simulink model "ofdm_txrx_supermimo_coded"  (Simulink 7.7)
settings: Solver=VariableStepDiscrete  SolverMode=SingleTasking  StartTime=0.0  StopTime=2*simOnly_simLength  RelTol=1e-3
PostLoadFcn:
  | ofdm_rx_supermimo_init
InitFcn:
  | ofdm_rx_supermimo_init
StartFcn:
  | ofdm_rx_supermimo_init
values from neighbouring files (symbols this model uses):
  ActionID_AFTransmit = 31    [ofdm_tx_supermimo_init.m]
  ActionID_Disabled = 0    [ofdm_tx_supermimo_init.m]
  ActionID_SetFlagA = 62    [ofdm_tx_supermimo_init.m]
  ActionID_SetFlagB = 61    [ofdm_tx_supermimo_init.m]
  BER_RAM_init_size = 2048    [ofdm_tx_supermimo_init.m]
  channel_h = [1 0; 1 0] * 0.3    [ofdm_tx_supermimo_init.m]
  CPLength = 16    [ofdm_tx_supermimo_init.m]
  longCorr_coef_i = [-4*real(LTS) 0]    [ofdm_rx_supermimo_init.m]
  longCorr_coef_q = [4*imag(LTS) 0]    [ofdm_rx_supermimo_init.m]
  max_CP_length = 16    [ofdm_tx_supermimo_init.m]
  max_num_baseRateSymbols = 31    [ofdm_tx_supermimo_init.m]
  max_num_subcarriers = 64    [ofdm_tx_supermimo_init.m]
  max_num_trainingSymbols = 15    [ofdm_tx_supermimo_init.m]
  max_numBytes = 16384    [ofdm_tx_supermimo_init.m]
  max_OFDM_symbols = 1023    [ofdm_tx_supermimo_init.m]
  modConstellation_qam16 = .75*[1 3 -1 -3]./3    [ofdm_tx_supermimo_init.m]
  modConstellation_qam64 = 0.875*[3 1 5 7 -3 -1 -5 -7]./7    [ofdm_tx_supermimo_init.m]
  modConstellation_qpsk = [1 -1]./sqrt(2)    [ofdm_tx_supermimo_init.m]
  numBaseRateSymbols = numBaseRateSymbols *2    [ofdm_tx_supermimo_init.m]
  numSubcarriers = 64    [ofdm_tx_supermimo_init.m]
  numTrainingSymbols = 2    [ofdm_tx_supermimo_init.m]
  pktTiming_count_payloadStart = pktTiming_count_lsStart + pktTiming_ls_num*pktTiming_ls_length    [ofdm_rx_supermimo_init.m]
  preamble = preamble_scale*[shortsyms_10 longsyms_2]    [ofdm_tx_supermimo_init.m]
  preamble_ROM = preamble_I_ROM + preamble_Q_ROM*2^16    [ofdm_tx_supermimo_init.m]
  RAM_init_size = max_numBytes    (= 16384)    [ofdm_tx_supermimo_init.m]
  RxDataScrambling_Seq = [40 198 78 63 82 173 102 245 48 111 172 115 147 230 216 93 72 65 62 2 205 242 122 90 128 83 105 97 73 10 5 252]    [ofdm_tx_supermimo_init.m]
  shortSymbol_time = shortSymbol_time(1:16).'    [ofdm_tx_supermimo_init.m]
  simOnly_numSamples = length(preamble)+( (numSubcarriers+CPLength)*(numTrainingSymbols + numBaseRateSymbols + numFullRateSymbols/2) )    [ofdm_tx_supermimo_init.m]
  train = [train train]    [ofdm_tx_supermimo_init.m]
  TxDataScrambling_Seq = [40 198 78 63 82 173 102 245 48 111 172 115 147 230 216 93 72 65 62 2 205 242 122 90 128 83 105 97 73 10 5 252]    [ofdm_tx_supermimo_init.m]
undefined symbols (not defined in the file or in its folder): AntA, AntB, BaseRate, Down, gcbh, numBuffs, numBytes, Up, xlBlockLoadCallback, xlGetNormalizedPeriod, xlipgetpartsetting
libraries: xbsIndex_r4 x5430
(part 36 of 49)

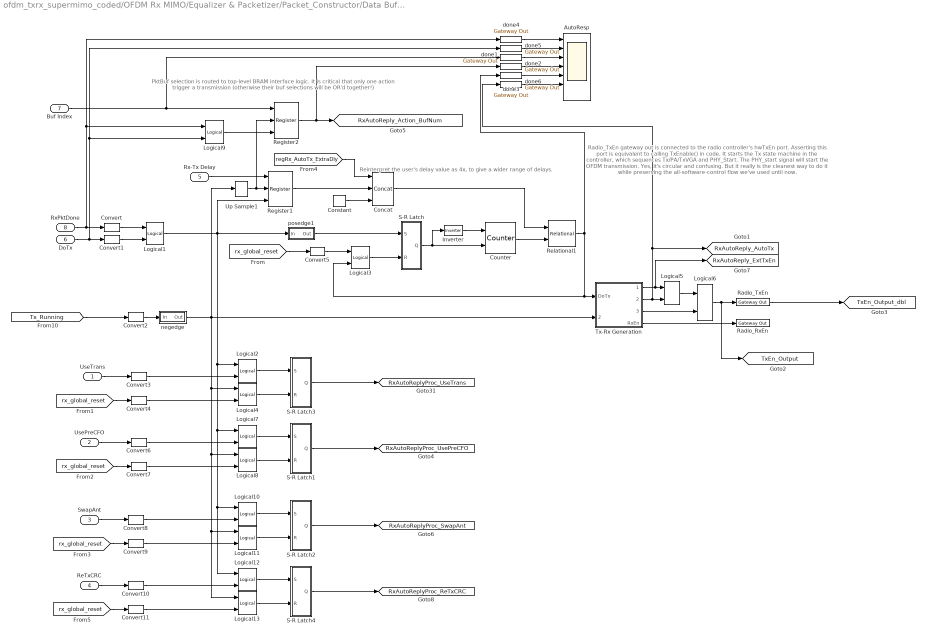
== system ofdm_txrx_supermimo_coded/OFDM Rx MIMO/Equalizer & Packetizer/Packet_Constructor/Data Buffer/AutoResponse Processing/AutoResponse I/O ==
  note: Radio_TxEn gateway out is connected to the radio controller's hwTxEn port.
      | Asserting this port is equivalent to calling TxEnable() in code. It starts the Tx
      | state machine in the controller, which sequences Tx/PA/TxVGA and PHY_Start.
      | The PHY_start signal will start the OFDM transmission.
      |
      | Yes, it's circular and confusing. But it really is the cleanest way to do it while
      | preserving the all-software-control flow we've used until now.
  note: PktBuf selection is routed to top-level
      | BRAM interface logic. It is critical that only
      | one action trigger a transmission (otherwise
      | their buf selections will be OR'd together!)
  note: Reinterpret the user's delay value as 4x,
      | to give a wider range of delays.
  UseTrans : Inport
  UsePreCFO : Inport  Port=2
  SwapAnt : Inport  Port=3
  ReTxCRC : Inport  Port=4
  Rx-Tx Delay : Inport  Port=5
  DoTx : Inport  Port=6
  Buf Index : Inport  Port=7
  RxPktDone : Inport  Port=8
  AutoResp : Scope  NumInputPorts=7
      settings: TimeRange=392.7801724137931  YMin=-0.5~-0.25~-1~-1~0~0~0  YMax=0.5~0.15~1~1~1~3500~1  SaveName=ScopeData25
  Concat : xbsIndex_r4/Concat  num_inputs=3  dbl_ovrd=off  block_type=concat
      settings: block_version=10.1.3
  Constant : xbsIndex_r4/Constant  const=0  gui_display_data_type=Fixed-point  arith_type=Unsigned  n_bits=1  bin_pt=0  preci_type=Single  exp_width=8  frac_width=24  explicit_period=off  period=1  equ=P=C  opselect=C  inp2=PCIN>>17  opr=+  inp1=P  carry=CIN  dbl_ovrd=off  block_type=constant
  Convert{,1..11} (12 alike) : xbsIndex_r4/Convert  gui_display_data_type=Fixed-point  arith_type=Boolean  n_bits=16  bin_pt=14  float_type=Single  exp_bits=8  fraction_bits=24  quantization=Truncate  overflow=Wrap  en=off  latency=0  dbl_ovrd=off  pipeline=off  block_type=convert
      settings: block_version=10.1.3
  Counter : xbsIndex_r4/Counter  cnt_type=Free Running  cnt_to=0  operation=Up  start_count=0  cnt_by_val=1  arith_type=Unsigned  n_bits=14  bin_pt=0  load_pin=off  rst=on  en=on  explicit_period=off  period=4  dbl_ovrd=off  use_behavioral_HDL=off  implementation=Fabric  use_rpm=off  block_type=counter
      settings: block_version=VER_STRING_GOES_HERE
  Inverter : xbsIndex_r4/Inverter  en=off  latency=0  dbl_ovrd=off  block_type=inv
      settings: xl_area=[0 0 0 0 0 0 0]  block_version=8.2
  Logical{1..2,7,9..10,12} (6 alike) : xbsIndex_r4/Logical  logical_function=AND  inputs=2  en=off  latency=0  precision=Full  arith_type=Unsigned  n_bits=16  bin_pt=0  align_bp=on  dbl_ovrd=off  block_type=logical
  Logical{3..6,8,11,13} (7 alike) : xbsIndex_r4/Logical  logical_function=OR  inputs=2  en=off  latency=0  precision=Full  arith_type=Unsigned  n_bits=16  bin_pt=0  align_bp=on  dbl_ovrd=off  block_type=logical
  Radio_RxEn : xbsIndex_r4/Gateway Out  inherit_from_input=off  hdl_port=on  timing_constraint=None  locs_specified=off  LOCs={}  UseAsDAC=off  DACChannel='1'  block_type=gatewayout
      settings: infoedit="Gateway out block.  Converts Xilinx fixed point inputs into ouputs of type Simulink integer, double, or fixed point.<P><P>Hardware notes:  In hardware these blocks become top level output ports or are discarded, depending on how they are configured."  block_version=10.1.3
  Radio_TxEn : xbsIndex_r4/Gateway Out  inherit_from_input=off  hdl_port=on  timing_constraint=None  locs_specified=off  LOCs={}  UseAsDAC=off  DACChannel='1'  block_type=gatewayout
      settings: infoedit="Gateway out block.  Converts Xilinx fixed point inputs into ouputs of type Simulink integer, double, or fixed point.<P><P>Hardware notes:  In hardware these blocks become top level output ports or are discarded, depending on how they are configured."  block_version=10.1.3
  Register1 : xbsIndex_r4/Register  init=0  rst=on  en=on  dbl_ovrd=off  block_type=register
      settings: xl_area=[5 10 0 0 0 0 0]  block_version=VER_STRING_GOES_HERE
  Register2 : xbsIndex_r4/Register  init=0  rst=on  en=on  dbl_ovrd=off  block_type=register
      settings: xl_area=[5 10 0 0 0 0 0]  block_version=VER_STRING_GOES_HERE
  Relational1 : xbsIndex_r4/Relational  mode=a<b  en=off  latency=0  dbl_ovrd=off  block_type=relational
      settings: block_version=VER_STRING_GOES_HERE
  S-R Latch : Subsystem
  S-R Latch1 : Subsystem
  S-R Latch2 : Subsystem
  S-R Latch3 : Subsystem
  S-R Latch4 : Subsystem
  Tx-Rx Generation : Subsystem
  Up Sample1 : xbsIndex_r4/Up Sample  sample_ratio=2  copy_samples=on  en=off  latency=0  dbl_ovrd=off  block_type=usamp
      settings: infoedit="Up samples input data.  Inserted values  can be zeros or copies of the most recent input sample.<P><P>Hardware notes: No hardware is needed if inserted values are copies of the input sample; otherwise, a mux and single bit flip-flop are used."  block_version=10.1.3
  done{1..6} (6 alike) : xbsIndex_r4/Gateway Out  inherit_from_input=off  hdl_port=off  timing_constraint=None  locs_specified=off  LOCs={}  UseAsDAC=off  DACChannel='1'  block_type=gatewayout
      settings: infoedit="Gateway out block.  Converts Xilinx fixed point inputs into ouputs of type Simulink integer, double, or fixed point.<P><P>Hardware notes:  In hardware these blocks become top level output ports or are discarded, depending on how they are configured."  xl_area=[0 0 0 0 1 0 0]  block_version=VER_STRING_GOES_HERE
  negedge : Subsystem
  posedge1 : Subsystem
  tag scope: RxAutoReplyProc_ReTxCRC=global, RxAutoReplyProc_SwapAnt=global, RxAutoReplyProc_UsePreCFO=global, RxAutoReplyProc_UseTrans=global, RxAutoReply_Action_BufNum=global, RxAutoReply_AutoTx=global, RxAutoReply_ExtTxEn=global, TxEn_Output=global, TxEn_Output_dbl=global
  wires:
  S-R Latch/1(Q) -> Counter/2, Inverter/1
  negedge/1(Out) -> Up Sample1/1, Logical4/1, Logical8/1, Logical11/1, Logical13/1, Tx-Rx Generation/2(TxDone)
  [Tx_Running] -> Convert2/1
  posedge1/1(Out) -> S-R Latch/1(S)
  Inverter/1 -> Counter/1
  Tx-Rx Generation/4(RxEn) -> Radio_RxEn/1
  Relational1/1 -> Logical3/2, Tx-Rx Generation/1(DoTx), done3/1
  Logical3/1 -> S-R Latch/2(R)
  [rx_global_reset] -> Convert5/1
  Logical1/1 -> posedge1/1(In), Register1/3, Logical2/1, Logical7/1, Logical10/1, Logical12/1
  Logical2/1 -> S-R Latch3/1(S)
  S-R Latch3/1(Q) -> [RxAutoReplyProc_UseTrans]
  Radio_TxEn/1 -> [TxEn_Output_dbl]
  DoTx/1 -> Convert1/1, Logical9/2, done5/1
  RxPktDone/1 -> Convert/1, Logical9/1, done4/1
  UseTrans/1 -> Convert3/1
  [rx_global_reset] -> Convert4/1
  Logical4/1 -> S-R Latch3/2(R)
  Convert/1 -> Logical1/1
  Convert1/1 -> Logical1/2
  Convert2/1 -> negedge/1(In)
  Convert3/1 -> Logical2/2
  Convert4/1 -> Logical4/2
  Convert5/1 -> Logical3/1
  Tx-Rx Generation/2(AutoTxEn) -> Logical5/2, [RxAutoReply_AutoTx], done6/1
  Logical6/1 -> Radio_TxEn/1, [TxEn_Output]
  Tx-Rx Generation/3(UserTxEn) -> Logical6/2
  Tx-Rx Generation/1(ExtTxEn) -> Logical5/1, [RxAutoReply_ExtTxEn]
  Logical5/1 -> Logical6/1
  Rx-Tx Delay/1 -> Register1/1
  Up Sample1/1 -> Register1/2, Register2/2
  Logical7/1 -> S-R Latch1/1(S)
  S-R Latch1/1(Q) -> [RxAutoReplyProc_UsePreCFO]
  UsePreCFO/1 -> Convert6/1
  [rx_global_reset] -> Convert7/1
  Logical8/1 -> S-R Latch1/2(R)
  Convert6/1 -> Logical7/2
  Convert7/1 -> Logical8/2
  Logical9/1 -> Register2/3
  Buf Index/1 -> Register2/1, done1/1
  Register2/1 -> [RxAutoReply_Action_BufNum], done2/1
  Constant/1 -> Concat/3
  Register1/1 -> Concat/2
  Concat/1 -> Relational1/1
  Counter/1 -> Relational1/2
  SwapAnt/1 -> Convert8/1
  Logical10/1 -> S-R Latch2/1(S)
  Logical11/1 -> S-R Latch2/2(R)
  Convert8/1 -> Logical10/2
  [rx_global_reset] -> Convert9/1
  Convert9/1 -> Logical11/2
  S-R Latch2/1(Q) -> [RxAutoReplyProc_SwapAnt]
  [regRx_AutoTx_ExtraDly] -> Concat/1
  done4/1 -> AutoResp/1
  done5/1 -> AutoResp/2
  done1/1 -> AutoResp/3
  done2/1 -> AutoResp/4
  done3/1 -> AutoResp/5
  done6/1 -> AutoResp/6
  ReTxCRC/1 -> Convert10/1
  Logical12/1 -> S-R Latch4/1(S)
  Logical13/1 -> S-R Latch4/2(R)
  Convert10/1 -> Logical12/2
  [rx_global_reset] -> Convert11/1
  Convert11/1 -> Logical13/2
  S-R Latch4/1(Q) -> [RxAutoReplyProc_ReTxCRC]

== system ofdm_txrx_supermimo_coded/OFDM Rx MIMO/Equalizer & Packetizer/Packet_Constructor/Data Buffer/AutoResponse Processing/Flag A ==
  note: The flag is cleared after every packet reception. The clear is
      | delayed 2 cycles so the matching logic can use the flag set by the
      | previous packet in combination with header/status values
      | from the packet that just finished.
      |
      | The flag is set 1 cycle later if some action asserts the SetFlag input.
  SetFlag : Inport
  PktDone : Inport  Port=2
  Delay : xbsIndex_r4/Delay  rst=off  infoeditControl=Selection of Reset will increase slice count due to use of real FFs and instead of SRLs  en=off  latency=2  dbl_ovrd=off  reg_retiming=off  block_type=delay
  Delay1 : xbsIndex_r4/Delay  rst=off  infoeditControl=Selection of Reset will increase slice count due to use of real FFs and instead of SRLs  en=off  latency=1  dbl_ovrd=off  reg_retiming=off  block_type=delay
  Flag A Latch : Subsystem
  Logical1 : xbsIndex_r4/Logical  logical_function=AND  inputs=2  en=off  latency=0  precision=Full  arith_type=Unsigned  n_bits=16  bin_pt=0  align_bp=on  dbl_ovrd=off  block_type=logical
  Logical2 : xbsIndex_r4/Logical  logical_function=OR  inputs=3  en=off  latency=0  precision=Full  arith_type=Unsigned  n_bits=16  bin_pt=0  align_bp=on  dbl_ovrd=off  block_type=logical
  Flag : Outport
  wires:
  [rx_global_reset] -> Logical2/2
  Logical2/1 -> Flag A Latch/2(R)
  Delay/1 -> Logical2/1, Delay1/1
  Logical1/1 -> Flag A Latch/1(S)
  Flag A Latch/1(Q) -> Flag/1
  PktDone/1 -> Delay/1
  SetFlag/1 -> Logical1/1
  Delay1/1 -> Logical1/2
  [RxReg_ResetFlagA] -> Logical2/3

== system ofdm_txrx_supermimo_coded/OFDM Rx MIMO/Equalizer & Packetizer/Packet_Constructor/Data Buffer/AutoResponse Processing/Flag B ==
  note: The flag is cleared after every packet reception. The clear is
      | delayed 2 cycles so the matching logic can use the flag set by the
      | previous packet in combination with header/status values
      | from the packet that just finished.
      |
      | The flag is set 1 cycle later if some action asserts the SetFlag input.
  SetFlag : Inport
  PktDone : Inport  Port=2
  Delay : xbsIndex_r4/Delay  rst=off  infoeditControl=Selection of Reset will increase slice count due to use of real FFs and instead of SRLs  en=off  latency=2  dbl_ovrd=off  reg_retiming=off  block_type=delay
  Delay1 : xbsIndex_r4/Delay  rst=off  infoeditControl=Selection of Reset will increase slice count due to use of real FFs and instead of SRLs  en=off  latency=1  dbl_ovrd=off  reg_retiming=off  block_type=delay
  Flag B Latch : Subsystem
  Logical1 : xbsIndex_r4/Logical  logical_function=AND  inputs=2  en=off  latency=0  precision=Full  arith_type=Unsigned  n_bits=16  bin_pt=0  align_bp=on  dbl_ovrd=off  block_type=logical
  Logical2 : xbsIndex_r4/Logical  logical_function=OR  inputs=3  en=off  latency=0  precision=Full  arith_type=Unsigned  n_bits=16  bin_pt=0  align_bp=on  dbl_ovrd=off  block_type=logical
  Flag : Outport
  wires:
  [RxReg_ResetFlagB] -> Logical2/3
  Delay1/1 -> Logical1/2
  SetFlag/1 -> Logical1/1
  PktDone/1 -> Delay/1
  Flag B Latch/1(Q) -> Flag/1
  Logical1/1 -> Flag B Latch/1(S)
  Delay/1 -> Delay1/1, Logical2/1
  Logical2/1 -> Flag B Latch/2(R)
  [rx_global_reset] -> Logical2/2

== system ofdm_txrx_supermimo_coded/OFDM Rx MIMO/Equalizer & Packetizer/Packet_Constructor/Data Buffer/AutoResponse Processing/Header Byte Matching ==
  RxByteAddr : Inport
  RxByte : Inport  Port=2
  RxByteWE : Inport  Port=3
  Rst : Inport  Port=4
  Concat : xbsIndex_r4/Concat  num_inputs=8  dbl_ovrd=off  block_type=concat
      settings: block_version=10.1.3
  Constant : xbsIndex_r4/Constant  const=0  gui_display_data_type=Fixed-point  arith_type=Unsigned  n_bits=1  bin_pt=0  preci_type=Single  exp_width=8  frac_width=24  explicit_period=off  period=1  equ=P=C  opselect=C  inp2=PCIN>>17  opr=+  inp1=P  carry=CIN  dbl_ovrd=off  block_type=constant
  Field Slices : Subsystem
  Field Slices1 : Subsystem
  Field Slices2 : Subsystem
  Field Slices3 : Subsystem
  Field Slices4 : Subsystem
  Field Slices5 : Subsystem
  Match0 : Subsystem
  Match1 : Subsystem
  Match2 : Subsystem
  Match3 : Subsystem
  Match4 : Subsystem
  Match5 : Subsystem
  Match_8b : Outport
  wires:
  [regAutoReply_match5] -> Field Slices5/1(Reg 32b)
  Field Slices5/1(Addr) -> Match5/5(Match ByteAddr)
  Field Slices5/2(Len) -> Match5/6(Match Length)
  Field Slices5/3(Val) -> Match5/7(Match Bytes)
  RxByteAddr/1 -> Match0/2(Rx ByteAddr), Match1/2(Rx ByteAddr), Match2/2(Rx ByteAddr), Match3/2(Rx ByteAddr), Match4/2(Rx ByteAddr), Match5/2(Rx ByteAddr)
  RxByte/1 -> Match0/3(Rx Byte), Match1/3(Rx Byte), Match2/3(Rx Byte), Match3/3(Rx Byte), Match4/3(Rx Byte), Match5/3(Rx Byte)
  RxByteWE/1 -> Match0/4(Rx ByteVin), Match1/4(Rx ByteVin), Match2/4(Rx ByteVin), Match3/4(Rx ByteVin), Match4/4(Rx ByteVin), Match5/4(Rx ByteVin)
  Rst/1 -> Match0/1(Rst), Match1/1(Rst), Match2/1(Rst), Match3/1(Rst), Match4/1(Rst), Match5/1(Rst)
  [regAutoReply_match4] -> Field Slices4/1(Reg 32b)
  Field Slices4/1(Addr) -> Match4/5(Match ByteAddr)
  Field Slices4/2(Len) -> Match4/6(Match Length)
  Field Slices4/3(Val) -> Match4/7(Match Bytes)
  [regAutoReply_match3] -> Field Slices3/1(Reg 32b)
  [regAutoReply_match2] -> Field Slices2/1(Reg 32b)
  Field Slices3/1(Addr) -> Match3/5(Match ByteAddr)
  Field Slices3/2(Len) -> Match3/6(Match Length)
  Field Slices3/3(Val) -> Match3/7(Match Bytes)
  Field Slices2/1(Addr) -> Match2/5(Match ByteAddr)
  Field Slices2/2(Len) -> Match2/6(Match Length)
  Field Slices2/3(Val) -> Match2/7(Match Bytes)
  [regAutoReply_match1] -> Field Slices1/1(Reg 32b)
  [regAutoReply_match0] -> Field Slices/1(Reg 32b)
  Field Slices1/1(Addr) -> Match1/5(Match ByteAddr)
  Field Slices1/2(Len) -> Match1/6(Match Length)
  Field Slices1/3(Val) -> Match1/7(Match Bytes)
  Field Slices/1(Addr) -> Match0/5(Match ByteAddr)
  Field Slices/2(Len) -> Match0/6(Match Length)
  Field Slices/3(Val) -> Match0/7(Match Bytes)
  Match5/1(Match) -> Concat/3
  Match4/1(Match) -> Concat/4
  Match3/1(Match) -> Concat/5
  Match2/1(Match) -> Concat/6
  Match1/1(Match) -> Concat/7
  Match0/1(Match) -> Concat/8
  Concat/1 -> Match_8b/1
  Constant/1 -> Concat/1, Concat/2

== system ofdm_txrx_supermimo_coded/OFDM Rx MIMO/Equalizer & Packetizer/Packet_Constructor/Data Buffer/BER Calc/BER Pkt  Buffer ==
  Addr : Inport
  ByteComp : Scope  NumInputPorts=3
      settings: TimeRange=6000  YMin=0~0~22.5  YMax=32.5~250~55  SaveName=ScopeData80
  LSB : xbsIndex_r4/Slice  nbits=log2(BER_RAM_init_size)  boolean_output=off  mode=Lower Bit Location + Width  bit1=0  base1=MSB of Input  bit0=0  base0=LSB of Input  dbl_ovrd=off  block_type=slice
      settings: infoedit="Extracts a given range of bits from each input sample and presents it at the output.  The output type is ordinarily unsigned with binary point at zero, but can be Boolean when the slice is one bit wide.<P><P>Hardware notes: In hardware this block costs nothing."  block_version=8.2.01
  Mux : xbsIndex_r4/Mux  inputs=2  en=off  latency=0  precision=Full  arith_type=Unsigned  n_bits=16  bin_pt=14  quantization=Truncate  overflow=Wrap  dbl_ovrd=off  block_type=mux
      settings: block_version=10.1.2
  Read-only (non-PLB) BER Pkt Buffer : Subsystem
  Simulation Multiplexer : xbsIndex_r4/Simulation Multiplexer  sim_sel=1  hw_sel=2  block_type=simmux
      settings: infoedit="Distinguish input subsystems as \"simulation only\" and \"generation only\".  The input specified For Simulation will be used during Simulink simulation.  The input specified For Generation will be used during code generation.  This block will typically be used on the output of a HW Co-Simulation, Black Box, or ModelSim subsystem.<P><P>Hardware Notes: This block costs nothing."  block_version=10.1.3
  done1 : xbsIndex_r4/Gateway Out  inherit_from_input=off  hdl_port=off  timing_constraint=None  locs_specified=off  LOCs={}  UseAsDAC=off  DACChannel='1'  block_type=gatewayout
      settings: infoedit="Gateway out block.  Converts Xilinx fixed point inputs into ouputs of type Simulink integer, double, or fixed point.<P><P>Hardware notes:  In hardware these blocks become top level output ports or are discarded, depending on how they are configured."  xl_area=[0 0 0 0 1 0 0]  block_version=VER_STRING_GOES_HERE
  done2 : xbsIndex_r4/Gateway Out  inherit_from_input=off  hdl_port=off  timing_constraint=None  locs_specified=off  LOCs={}  UseAsDAC=off  DACChannel='1'  block_type=gatewayout
      settings: infoedit="Gateway out block.  Converts Xilinx fixed point inputs into ouputs of type Simulink integer, double, or fixed point.<P><P>Hardware notes:  In hardware these blocks become top level output ports or are discarded, depending on how they are configured."  xl_area=[0 0 0 0 1 0 0]  block_version=VER_STRING_GOES_HERE
  done8 : xbsIndex_r4/Gateway Out  inherit_from_input=off  hdl_port=off  timing_constraint=None  locs_specified=off  LOCs={}  UseAsDAC=off  DACChannel='1'  block_type=gatewayout
      settings: infoedit="Gateway out block.  Converts Xilinx fixed point inputs into ouputs of type Simulink integer, double, or fixed point.<P><P>Hardware notes:  In hardware these blocks become top level output ports or are discarded, depending on how they are configured."  xl_area=[0 0 0 0 1 0 0]  block_version=VER_STRING_GOES_HERE
  ByteOut : Outport
  wires:
  Addr/1 -> LSB/1
  LSB/1 -> Read-only (non-PLB) BER Pkt Buffer/1(Addr), done2/1
  Read-only (non-PLB) BER Pkt Buffer/1(Byte) -> Mux/2, done8/1
  [regRx_FlexBERMode] -> Mux/1
  Simulation Multiplexer/1 -> Mux/3, done1/1
  Mux/1 -> ByteOut/1
  done8/1 -> ByteComp/1
  done1/1 -> ByteComp/2
  done2/1 -> ByteComp/3
  [FlexBER_TxPktBuffBtyte_SIM] -> Simulation Multiplexer/1
  [FlexBER_TxPktBuffBtyte_HW] -> Simulation Multiplexer/2

== system ofdm_txrx_supermimo_coded/OFDM Rx MIMO/Equalizer & Packetizer/Packet_Constructor/Data Buffer/BER Calc/BER WEn ==
  note: Calculate the BER of all bytes except checksum bytes.
      | There are up to 6 checksum bytes:
      | Last two bytes of the header
      | Last four bytes of the paylaod IFF there are any payload bytes after the header
  BER_addr : Inport
  BER_val : Inport  Port=2
  numBytes : Inport  Port=3
  AddSub{1..3,6} (4 alike) : xbsIndex_r4/AddSub  mode=Subtraction  use_carryin=off  use_carryout=off  en=off  latency=0  precision=Full  arith_type=Unsigned  n_bits=8  bin_pt=2  quantization=Truncate  overflow=Wrap  dbl_ovrd=off  use_behavioral_HDL=on  hw_selection=Fabric  pipelined=off  use_rpm=on  block_type=addsub
      settings: xl_area=[0, 0, 0, 0, 0, 0, 0]  block_version=VER_STRING_GOES_HERE
  Constant : xbsIndex_r4/Constant  const=4  gui_display_data_type=Fixed-point  arith_type=Unsigned  n_bits=3  bin_pt=0  preci_type=Single  exp_width=8  frac_width=24  explicit_period=off  period=1  equ=P=C  opselect=C  inp2=PCIN>>17  opr=+  inp1=P  carry=CIN  dbl_ovrd=off  block_type=constant
      settings: block_version=VER_STRING_GOES_HERE
  Constant1 : xbsIndex_r4/Constant  const=2  gui_display_data_type=Fixed-point  arith_type=Unsigned  n_bits=2  bin_pt=0  preci_type=Single  exp_width=8  frac_width=24  explicit_period=off  period=1  equ=P=C  opselect=C  inp2=PCIN>>17  opr=+  inp1=P  carry=CIN  dbl_ovrd=off  block_type=constant
      settings: block_version=VER_STRING_GOES_HERE
  Constant2 : xbsIndex_r4/Constant  const=2  gui_display_data_type=Fixed-point  arith_type=Unsigned  n_bits=2  bin_pt=0  preci_type=Single  exp_width=8  frac_width=24  explicit_period=off  period=1  equ=P=C  opselect=C  inp2=PCIN>>17  opr=+  inp1=P  carry=CIN  dbl_ovrd=off  block_type=constant
      settings: block_version=VER_STRING_GOES_HERE
  Constant3 : xbsIndex_r4/Constant  const=1  gui_display_data_type=Fixed-point  arith_type=Unsigned  n_bits=2  bin_pt=0  preci_type=Single  exp_width=8  frac_width=24  explicit_period=off  period=1  equ=P=C  opselect=C  inp2=PCIN>>17  opr=+  inp1=P  carry=CIN  dbl_ovrd=off  block_type=constant
      settings: block_version=VER_STRING_GOES_HERE
  Inverter : xbsIndex_r4/Inverter  en=off  latency=0  dbl_ovrd=off  block_type=inv
      settings: xl_area=[0, 0, 0, 0, 0, 0, 0]  block_version=VER_STRING_GOES_HERE
  Inverter1 : xbsIndex_r4/Inverter  en=off  latency=0  dbl_ovrd=off  block_type=inv
      settings: xl_area=[0, 0, 0, 0, 0, 0, 0]  block_version=VER_STRING_GOES_HERE
  Inverter2 : xbsIndex_r4/Inverter  en=off  latency=0  dbl_ovrd=off  block_type=inv
      settings: xl_area=[0, 0, 0, 0, 0, 0, 0]  block_version=VER_STRING_GOES_HERE
  Logical1 : xbsIndex_r4/Logical  logical_function=AND  inputs=5  en=off  latency=0  precision=Full  arith_type=Unsigned  n_bits=1  bin_pt=0  align_bp=on  dbl_ovrd=off  block_type=logical
      settings: xl_area=[0, 0, 0, 0, 0, 0, 0]  block_version=VER_STRING_GOES_HERE
  Logical2 : xbsIndex_r4/Logical  logical_function=OR  inputs=2  en=off  latency=0  precision=Full  arith_type=Unsigned  n_bits=1  bin_pt=0  align_bp=on  dbl_ovrd=off  block_type=logical
      settings: xl_area=[0, 0, 0, 0, 0, 0, 0]  block_version=VER_STRING_GOES_HERE
  Logical3 : xbsIndex_r4/Logical  logical_function=AND  inputs=3  en=off  latency=0  precision=Full  arith_type=Unsigned  n_bits=1  bin_pt=0  align_bp=on  dbl_ovrd=off  block_type=logical
      settings: xl_area=[0, 0, 0, 0, 0, 0, 0]  block_version=VER_STRING_GOES_HERE
  Logical4 : xbsIndex_r4/Logical  logical_function=AND  inputs=2  en=off  latency=0  precision=Full  arith_type=Unsigned  n_bits=1  bin_pt=0  align_bp=on  dbl_ovrd=off  block_type=logical
      settings: xl_area=[0, 0, 0, 0, 0, 0, 0]  block_version=VER_STRING_GOES_HERE
  Logical8 : xbsIndex_r4/Logical  logical_function=AND  inputs=3  en=off  latency=0  precision=Full  arith_type=Unsigned  n_bits=1  bin_pt=0  align_bp=on  dbl_ovrd=off  block_type=logical
      settings: xl_area=[0, 0, 0, 0, 0, 0, 0]  block_version=VER_STRING_GOES_HERE
  Relational{,1..2,5} (4 alike) : xbsIndex_r4/Relational  mode=a>b  en=off  latency=0  dbl_ovrd=off  block_type=relational
      settings: xl_area=[0, 0, 0, 0, 0, 0, 0]  block_version=VER_STRING_GOES_HERE
  Relational3 : xbsIndex_r4/Relational  mode=a!=b  en=off  latency=0  dbl_ovrd=off  block_type=relational
      settings: xl_area=[0, 0, 0, 0, 0, 0, 0]  block_version=VER_STRING_GOES_HERE
  Relational4 : xbsIndex_r4/Relational  mode=a!=b  en=off  latency=0  dbl_ovrd=off  block_type=relational
      settings: xl_area=[0, 0, 0, 0, 0, 0, 0]  block_version=VER_STRING_GOES_HERE
  Rx Bytes : Scope  NumInputPorts=5
      settings: TimeRange=1800  YMin=0~50~24~15549.6~-1  YMax=1~275~30~17186.4~1  SaveName=ScopeData3
  done{1..2,6..7,9} (5 alike) : xbsIndex_r4/Gateway Out  inherit_from_input=off  hdl_port=off  timing_constraint=None  locs_specified=off  LOCs={}  UseAsDAC=off  DACChannel='1'  block_type=gatewayout
      settings: infoedit="Gateway out block.  Converts Xilinx fixed point inputs into ouputs of type Simulink integer, double, or fixed point.<P><P>Hardware notes:  In hardware these blocks become top level output ports or are discarded, depending on how they are configured."  xl_area=[0 0 0 0 1 0 0]  block_version=VER_STRING_GOES_HERE
  Vout : Outport
  wires:
  Inverter/1 -> Logical8/3
  BER_val/1 -> Logical8/2
  [rx_global_reset] -> Inverter/1
  Constant/1 -> AddSub6/2
  numBytes/1 -> AddSub6/1, Relational1/1, AddSub1/1, done6/1
  AddSub6/1 -> Relational/1
  BER_addr/1 -> Relational2/2, Relational/2, Relational5/2, Relational3/1, Relational4/1
  Logical8/1 -> Vout/1
  [regTxRx_numHeaderBytes] -> Relational1/2, AddSub2/1, AddSub3/1, Relational5/1, done7/1
  Relational/1 -> Logical1/2
  Relational1/1 -> Inverter1/1, Logical1/5
  Logical1/1 -> Logical2/1, done1/1
  AddSub1/1 -> Relational2/1
  Constant1/1 -> AddSub1/2
  Relational2/1 -> Logical3/3
  Inverter1/1 -> Logical3/2
  Logical3/1 -> Logical2/2
  Logical2/1 -> Logical8/1
  AddSub3/1 -> Relational3/2, done2/1
  AddSub2/1 -> Relational4/2, done9/1
  Relational3/1 -> Logical1/3
  Relational4/1 -> Logical1/4
  done6/1 -> Rx Bytes/1
  done7/1 -> Rx Bytes/2
  done1/1 -> Rx Bytes/3
  done9/1 -> Rx Bytes/5
  done2/1 -> Rx Bytes/4
  Constant3/1 -> AddSub3/2
  Constant2/1 -> AddSub2/2
  [regRx_BERignoreHeader] -> Logical4/1
  Relational5/1 -> Logical4/2
  Logical4/1 -> Inverter2/1
  Inverter2/1 -> Logical1/1, Logical3/1

== system ofdm_txrx_supermimo_coded/OFDM Rx MIMO/Equalizer & Packetizer/Packet_Constructor/Data Buffer/Descrambling & Pkt Buffer/BRAM WEn Decoding ==
  note: FlexBER mode configures the receiver to use
      | the Tx packet buffer as a "golden" packet for
      | BER calculations. In this mode, the receiver
      | does *not* write received packets to the pkt buffer!
  note: Two reasons to allow bytes to be written to the packet buffer:
      | -The PHY is in receive mode, and bytes are being generated by
      | the Rx pipeline.
      | OR
      | -The PHY is in transmit mode, generating random payloads interally
      | but wants to capture those payloads to a packet buffer for offline
      | analysis.
  Byte Addr : Inport
  WrEn : Inport  Port=2
  3LSB : xbsIndex_r4/Slice  nbits=3  boolean_output=off  mode=Lower Bit Location + Width  bit1=0  base1=MSB of Input  bit0=0  base0=LSB of Input  dbl_ovrd=off  block_type=slice
      settings: infoedit="Extracts a given range of bits from each input sample and presents it at the output.  The output type is ordinarily unsigned with binary point at zero, but can be Boolean when the slice is one bit wide.<P><P>Hardware notes: In hardware this block costs nothing."  block_version=8.2.02
  Concat : xbsIndex_r4/Concat  num_inputs=8  dbl_ovrd=off  block_type=concat
      settings: block_version=8.2.02
  Constant1 : xbsIndex_r4/Constant  const=0  gui_display_data_type=Fixed-point  arith_type=Unsigned  n_bits=3  bin_pt=0  preci_type=Single  exp_width=8  frac_width=24  explicit_period=off  period=2  equ=P=C  opselect=C  inp2=PCIN>>17  opr=+  inp1=P  carry=CIN  dbl_ovrd=off  block_type=constant
      settings: block_version=8.2.02
  Constant2 : xbsIndex_r4/Constant  const=1  gui_display_data_type=Fixed-point  arith_type=Unsigned  n_bits=3  bin_pt=0  preci_type=Single  exp_width=8  frac_width=24  explicit_period=off  period=2  equ=P=C  opselect=C  inp2=PCIN>>17  opr=+  inp1=P  carry=CIN  dbl_ovrd=off  block_type=constant
      settings: block_version=8.2.02
  Constant3 : xbsIndex_r4/Constant  const=2  gui_display_data_type=Fixed-point  arith_type=Unsigned  n_bits=3  bin_pt=0  preci_type=Single  exp_width=8  frac_width=24  explicit_period=off  period=2  equ=P=C  opselect=C  inp2=PCIN>>17  opr=+  inp1=P  carry=CIN  dbl_ovrd=off  block_type=constant
      settings: block_version=8.2.02
  Constant4 : xbsIndex_r4/Constant  const=3  gui_display_data_type=Fixed-point  arith_type=Unsigned  n_bits=3  bin_pt=0  preci_type=Single  exp_width=8  frac_width=24  explicit_period=off  period=2  equ=P=C  opselect=C  inp2=PCIN>>17  opr=+  inp1=P  carry=CIN  dbl_ovrd=off  block_type=constant
      settings: block_version=8.2.02
  Constant5 : xbsIndex_r4/Constant  const=4  gui_display_data_type=Fixed-point  arith_type=Unsigned  n_bits=3  bin_pt=0  preci_type=Single  exp_width=8  frac_width=24  explicit_period=off  period=2  equ=P=C  opselect=C  inp2=PCIN>>17  opr=+  inp1=P  carry=CIN  dbl_ovrd=off  block_type=constant
      settings: block_version=8.2.02
  Constant6 : xbsIndex_r4/Constant  const=5  gui_display_data_type=Fixed-point  arith_type=Unsigned  n_bits=3  bin_pt=0  preci_type=Single  exp_width=8  frac_width=24  explicit_period=off  period=2  equ=P=C  opselect=C  inp2=PCIN>>17  opr=+  inp1=P  carry=CIN  dbl_ovrd=off  block_type=constant
      settings: block_version=8.2.02
  Constant7 : xbsIndex_r4/Constant  const=6  gui_display_data_type=Fixed-point  arith_type=Unsigned  n_bits=3  bin_pt=0  preci_type=Single  exp_width=8  frac_width=24  explicit_period=off  period=2  equ=P=C  opselect=C  inp2=PCIN>>17  opr=+  inp1=P  carry=CIN  dbl_ovrd=off  block_type=constant
      settings: block_version=8.2.02
  Constant8 : xbsIndex_r4/Constant  const=7  gui_display_data_type=Fixed-point  arith_type=Unsigned  n_bits=3  bin_pt=0  preci_type=Single  exp_width=8  frac_width=24  explicit_period=off  period=2  equ=P=C  opselect=C  inp2=PCIN>>17  opr=+  inp1=P  carry=CIN  dbl_ovrd=off  block_type=constant
      settings: block_version=8.2.02
  Convert{,1..2,5} (4 alike) : xbsIndex_r4/Convert  gui_display_data_type=Fixed-point  arith_type=Unsigned  n_bits=1  bin_pt=0  float_type=Single  exp_bits=8  fraction_bits=24  quantization=Truncate  overflow=Wrap  en=off  latency=0  dbl_ovrd=off  pipeline=off  block_type=convert
      settings: block_version=10.1.3
  Convert4 : xbsIndex_r4/Convert  gui_display_data_type=Fixed-point  arith_type=Boolean  n_bits=1  bin_pt=0  float_type=Single  exp_bits=8  fraction_bits=24  quantization=Truncate  overflow=Wrap  en=off  latency=0  dbl_ovrd=off  pipeline=off  block_type=convert
      settings: block_version=10.1.3
  Inverter : xbsIndex_r4/Inverter  en=off  latency=0  dbl_ovrd=off  block_type=inv
  Inverter1 : xbsIndex_r4/Inverter  en=off  latency=0  dbl_ovrd=off  block_type=inv
  Inverter2 : xbsIndex_r4/Inverter  en=off  latency=0  dbl_ovrd=off  block_type=inv
  Logical{,1..7} (8 alike) : xbsIndex_r4/Logical  logical_function=AND  inputs=2  en=off  latency=0  precision=Full  arith_type=Unsigned  n_bits=16  bin_pt=0  align_bp=on  dbl_ovrd=off  block_type=logical
      settings: block_version=8.2.02
  Logical10 : xbsIndex_r4/Logical  logical_function=OR  inputs=2  en=off  latency=0  precision=Full  arith_type=Unsigned  n_bits=16  bin_pt=0  align_bp=on  dbl_ovrd=off  block_type=logical
  Logical8 : xbsIndex_r4/Logical  logical_function=AND  inputs=4  en=off  latency=0  precision=Full  arith_type=Unsigned  n_bits=16  bin_pt=0  align_bp=on  dbl_ovrd=off  block_type=logical
  Logical9 : xbsIndex_r4/Logical  logical_function=AND  inputs=4  en=off  latency=0  precision=Full  arith_type=Unsigned  n_bits=16  bin_pt=0  align_bp=on  dbl_ovrd=off  block_type=logical
  Relational{,1..7} (8 alike) : xbsIndex_r4/Relational  mode=a=b  en=off  latency=0  dbl_ovrd=off  block_type=relational
      settings: block_version=8.2.02
  Up Sample1 : xbsIndex_r4/Up Sample  sample_ratio=2  copy_samples=on  en=off  latency=0  dbl_ovrd=off  block_type=usamp
      settings: infoedit="Up samples input data.  Inserted values  can be zeros or copies of the most recent input sample.<P><P>Hardware notes: No hardware is needed if inserted values are copies of the input sample; otherwise, a mux and single bit flip-flop are used."  block_version=8.2.02
  Up Sample2 : xbsIndex_r4/Up Sample  sample_ratio=4  copy_samples=on  en=off  latency=0  dbl_ovrd=off  block_type=usamp
      settings: infoedit="Up samples input data.  Inserted values  can be zeros or copies of the most recent input sample.<P><P>Hardware notes: No hardware is needed if inserted values are copies of the input sample; otherwise, a mux and single bit flip-flop are used."  block_version=8.2.02
  Up Sample3 : xbsIndex_r4/Up Sample  sample_ratio=4  copy_samples=on  en=off  latency=0  dbl_ovrd=off  block_type=usamp
      settings: infoedit="Up samples input data.  Inserted values  can be zeros or copies of the most recent input sample.<P><P>Hardware notes: No hardware is needed if inserted values are copies of the input sample; otherwise, a mux and single bit flip-flop are used."  block_version=8.2.02
  Up Sample4 : xbsIndex_r4/Up Sample  sample_ratio=2  copy_samples=on  en=off  latency=0  dbl_ovrd=off  block_type=usamp
      settings: infoedit="Up samples input data.  Inserted values  can be zeros or copies of the most recent input sample.<P><P>Hardware notes: No hardware is needed if inserted values are copies of the input sample; otherwise, a mux and single bit flip-flop are used."  block_version=8.2.02
  Up Sample5 : xbsIndex_r4/Up Sample  sample_ratio=2  copy_samples=on  en=off  latency=0  dbl_ovrd=off  block_type=usamp
      settings: infoedit="Up samples input data.  Inserted values  can be zeros or copies of the most recent input sample.<P><P>Hardware notes: No hardware is needed if inserted values are copies of the input sample; otherwise, a mux and single bit flip-flop are used."  block_version=8.2.02
  ByteEn : Outport
  wires:
  Constant1/1 -> Relational/2
  Constant2/1 -> Relational1/2
  Constant3/1 -> Relational2/2
  Constant4/1 -> Relational3/2
  Constant5/1 -> Relational4/2
  Constant6/1 -> Relational5/2
  Constant7/1 -> Relational6/2
  Constant8/1 -> Relational7/2
  Byte Addr/1 -> 3LSB/1
  Relational/1 -> Logical/1
  Relational1/1 -> Logical1/1
  Relational2/1 -> Logical2/1
  Relational3/1 -> Logical3/1
  Relational4/1 -> Logical4/1
  Relational5/1 -> Logical5/1
  Relational6/1 -> Logical6/1
  Relational7/1 -> Logical7/1
  Concat/1 -> ByteEn/1
  3LSB/1 -> Relational/1, Relational1/1, Relational2/1, Relational3/1, Relational4/1, Relational5/1, Relational6/1, Relational7/1
  Logical9/1 -> Logical10/1
  Logical/1 -> Concat/1
  Logical1/1 -> Concat/2
  Logical2/1 -> Concat/3
  Logical3/1 -> Concat/4
  Logical4/1 -> Concat/5
  Logical5/1 -> Concat/6
  Logical6/1 -> Concat/7
  Logical7/1 -> Concat/8
  [regRx_FlexBERMode] -> Inverter/1
  Inverter/1 -> Up Sample1/1
  [regTx_PktRunning] -> Inverter2/1
  Inverter2/1 -> Logical9/3
  [Tx_Running] -> Inverter1/1
  Inverter1/1 -> Up Sample2/1
  Convert/1 -> Logical9/2
  Up Sample1/1 -> Convert1/1
  WrEn/1 -> Convert2/1
  Up Sample2/1 -> Convert/1
  Convert1/1 -> Logical9/1
  Convert4/1 -> Logical/2, Logical1/2, Logical2/2, Logical3/2, Logical4/2, Logical5/2, Logical6/2, Logical7/2
  Logical10/1 -> Convert4/1
  Logical8/1 -> Logical10/2
  Convert2/1 -> Logical9/4, Logical8/1
  [regTx_CaptureRandomPayload] -> Up Sample4/1
  [regTx_RandomPayload] -> Up Sample5/1
  [Tx_Running] -> Up Sample3/1
  Convert5/1 -> Logical8/2
  Up Sample3/1 -> Convert5/1
  Up Sample4/1 -> Logical8/3
  Up Sample5/1 -> Logical8/4

== system ofdm_txrx_supermimo_coded/OFDM Rx MIMO/Equalizer & Packetizer/Packet_Constructor/Data Buffer/Descrambling & Pkt Buffer/Data Src Sel ==
  note: When the Tx is in random payload mode, this block can
      | capture the internally generated payload and write it
      | to a packet buffer. The MAC can then dump it over
      | the wire for offline comparison to received packets.
  Addr : Inport
  Data : Inport  Port=2
  We : Inport  Port=3
  Convert : xbsIndex_r4/Convert  gui_display_data_type=Fixed-point  arith_type=Boolean  n_bits=16  bin_pt=14  float_type=Single  exp_bits=8  fraction_bits=24  quantization=Truncate  overflow=Wrap  en=off  latency=0  dbl_ovrd=off  pipeline=off  block_type=convert
      settings: block_version=10.1.3
  Convert1 : xbsIndex_r4/Convert  gui_display_data_type=Fixed-point  arith_type=Boolean  n_bits=16  bin_pt=14  float_type=Single  exp_bits=8  fraction_bits=24  quantization=Truncate  overflow=Wrap  en=off  latency=0  dbl_ovrd=off  pipeline=off  block_type=convert
      settings: block_version=10.1.3
  Convert2 : xbsIndex_r4/Convert  gui_display_data_type=Fixed-point  arith_type=Boolean  n_bits=16  bin_pt=14  float_type=Single  exp_bits=8  fraction_bits=24  quantization=Truncate  overflow=Wrap  en=off  latency=0  dbl_ovrd=off  pipeline=off  block_type=convert
      settings: block_version=10.1.3
  Logical7 : xbsIndex_r4/Logical  logical_function=AND  inputs=3  en=off  latency=0  precision=Full  arith_type=Unsigned  n_bits=16  bin_pt=0  align_bp=on  dbl_ovrd=off  block_type=logical
      settings: block_version=8.2.02
  Mux : xbsIndex_r4/Mux  inputs=2  en=off  latency=0  precision=Full  arith_type=Unsigned  n_bits=16  bin_pt=14  quantization=Truncate  overflow=Wrap  dbl_ovrd=off  block_type=mux
      settings: block_version=8.2.02
  Mux1 : xbsIndex_r4/Mux  inputs=2  en=off  latency=0  precision=Full  arith_type=Unsigned  n_bits=16  bin_pt=14  quantization=Truncate  overflow=Wrap  dbl_ovrd=off  block_type=mux
      settings: block_version=8.2.02
  Mux2 : xbsIndex_r4/Mux  inputs=2  en=off  latency=0  precision=Full  arith_type=Unsigned  n_bits=16  bin_pt=14  quantization=Truncate  overflow=Wrap  dbl_ovrd=off  block_type=mux
      settings: block_version=8.2.02
  Up Sample1 : xbsIndex_r4/Up Sample  sample_ratio=2  copy_samples=on  en=off  latency=0  dbl_ovrd=off  block_type=usamp
      settings: infoedit="Up samples input data.  Inserted values  can be zeros or copies of the most recent input sample.<P><P>Hardware notes: No hardware is needed if inserted values are copies of the input sample; otherwise, a mux and single bit flip-flop are used."  block_version=8.2.02
  " Addr" : Outport
  " Data" : Outport  Port=2
  " We" : Outport  Port=3
  wires:
  [regTx_CaptureRandomPayload] -> Convert1/1
  [regTx_RandomPayload] -> Convert2/1
  [Tx_Running] -> Convert/1
  Logical7/1 -> Mux/1, Mux1/1, Mux2/1
  [Tx_RandomPayloadByte] -> Mux/3
  Data/1 -> Mux/2
  Addr/1 -> Mux1/2
  [Tx_RandomPayloadByte_addr] -> Mux1/3
  [Tx_RandomPayloadByte_valid] -> Mux2/3
  We/1 -> Mux2/2
  Mux2/1 -> " We"/1
  Mux1/1 -> " Addr"/1
  Mux/1 -> " Data"/1
  Convert/1 -> Up Sample1/1
  Convert1/1 -> Logical7/2
  Convert2/1 -> Logical7/3
  Up Sample1/1 -> Logical7/1

== system ofdm_txrx_supermimo_coded/OFDM Rx MIMO/Equalizer & Packetizer/Packet_Constructor/Data Buffer/Descrambling & Pkt Buffer/DataReplication ==
  ByteIn : Inport
  Concat : xbsIndex_r4/Concat  num_inputs=8  dbl_ovrd=off  block_type=concat
      settings: block_version=8.2.02
  8BytesOut : Outport
  wires:
  ByteIn/1 -> Concat/1, Concat/2, Concat/3, Concat/4, Concat/5, Concat/6, Concat/7, Concat/8
  Concat/1 -> 8BytesOut/1

== system ofdm_txrx_supermimo_coded/OFDM Rx MIMO/Equalizer & Packetizer/Packet_Constructor/Data Buffer/Descrambling & Pkt Buffer/De-Scrambling ==
  Addr : Inport
  Data : Inport  Port=2
  We : Inport  Port=3
  5LSB : xbsIndex_r4/Slice  nbits=ceil(log2(length(RxDataScrambling_Seq)))  boolean_output=off  mode=Lower Bit Location + Width  bit1=0  base1=MSB of Input  bit0=0  base0=LSB of Input  dbl_ovrd=off  block_type=slice
      settings: infoedit="Extracts a given range of bits from each input sample and presents it at the output.  The output type is ordinarily unsigned with binary point at zero, but can be Boolean when the slice is one bit wide.<P><P>Hardware notes: In hardware this block costs nothing."  block_version=8.2.02
  Delay : xbsIndex_r4/Delay  rst=off  infoeditControl=Selection of Reset will increase slice count due to use of real FFs and instead of SRLs  en=off  latency=1  dbl_ovrd=off  reg_retiming=off  block_type=delay
      settings: infoedit=Hardware notes: A delay line is a chain, each link of which is an SRL16 followed by a flip-flop. If register retiming is enabled, the delay line is a chain of flip-flops.  block_version=8.2.02
  Delay1 : xbsIndex_r4/Delay  rst=off  infoeditControl=Selection of Reset will increase slice count due to use of real FFs and instead of SRLs  en=off  latency=1  dbl_ovrd=off  reg_retiming=off  block_type=delay
      settings: infoedit=Hardware notes: A delay line is a chain, each link of which is an SRL16 followed by a flip-flop. If register retiming is enabled, the delay line is a chain of flip-flops.  block_version=8.2.02
  Delay2 : xbsIndex_r4/Delay  rst=off  infoeditControl=Selection of Reset will increase slice count due to use of real FFs and instead of SRLs  en=off  latency=1  dbl_ovrd=off  reg_retiming=off  block_type=delay
      settings: infoedit=Hardware notes: A delay line is a chain, each link of which is an SRL16 followed by a flip-flop. If register retiming is enabled, the delay line is a chain of flip-flops.  block_version=8.2.02
  Logical : xbsIndex_r4/Logical  logical_function=XOR  inputs=2  en=off  latency=0  precision=Full  arith_type=Unsigned  n_bits=16  bin_pt=0  align_bp=on  dbl_ovrd=off  block_type=logical
      settings: block_version=8.2.02
  ROM : xbsIndex_r4/ROM  depth=length(RxDataScrambling_Seq)  initVector=RxDataScrambling_Seq  distributed_mem=Distributed memory  rst=off  init_reg=0  en=off  latency=1  gui_display_data_type=Fixed-point  arith_type=Unsigned  n_bits=8  bin_pt=0  preci_type=Single  exp_width=8  frac_width=24  dbl_ovrd=off  optimize=Area  use_rpm=on  block_type=sprom
      settings: block_version=8.2.02
  " Addr" : Outport
  " Data" : Outport  Port=2
  " We" : Outport  Port=3
  wires:
  ROM/1 -> Logical/1
  5LSB/1 -> ROM/1
  Delay/1 -> Logical/2
  Data/1 -> Delay/1
  We/1 -> Delay1/1
  Addr/1 -> Delay2/1, 5LSB/1
  Delay2/1 -> " Addr"/1
  Logical/1 -> " Data"/1
  Delay1/1 -> " We"/1

== system ofdm_txrx_supermimo_coded/OFDM Rx MIMO/Equalizer & Packetizer/Packet_Constructor/Data Buffer/Descrambling & Pkt Buffer/Error Injector (Sim Only!) ==
  ByteAddr : Inport
  Data_I : Inport  Port=2
  Constant : xbsIndex_r4/Constant  const=17  gui_display_data_type=Fixed-point  arith_type=Unsigned  n_bits=8  bin_pt=0  preci_type=Single  exp_width=8  frac_width=24  explicit_period=on  period=2  equ=P=C  opselect=C  inp2=PCIN>>17  opr=+  inp1=P  carry=CIN  dbl_ovrd=off  block_type=constant
      settings: block_version=10.1.2
  Constant1 : xbsIndex_r4/Constant  const=30  gui_display_data_type=Fixed-point  arith_type=Unsigned  n_bits=8  bin_pt=0  preci_type=Single  exp_width=8  frac_width=24  explicit_period=off  period=1  equ=P=C  opselect=C  inp2=PCIN>>17  opr=+  inp1=P  carry=CIN  dbl_ovrd=off  block_type=constant
      settings: block_version=10.1.2
  Mux : xbsIndex_r4/Mux  inputs=2  en=off  latency=0  precision=Full  arith_type=Unsigned  n_bits=16  bin_pt=14  quantization=Truncate  overflow=Wrap  dbl_ovrd=off  block_type=mux
      settings: block_version=10.1.2
  Relational : xbsIndex_r4/Relational  mode=a=b  en=off  latency=0  dbl_ovrd=off  block_type=relational
      settings: block_version=10.1.2
  Data_O : Outport
  wires:
  Constant/1 -> Mux/3
  Constant1/1 -> Relational/2
  Relational/1 -> Mux/1
  ByteAddr/1 -> Relational/1
  Data_I/1 -> Mux/2
  Mux/1 -> Data_O/1

== system ofdm_txrx_supermimo_coded/OFDM Rx MIMO/Equalizer & Packetizer/Packet_Constructor/Data Buffer/Error Checking/CRC16 Calc ==
  ByteIn : Inport
  Vin : Inport  Port=2
  Rst : Inport  Port=3
  8LSB : xbsIndex_r4/Slice  nbits=8  boolean_output=off  mode=Lower Bit Location + Width  bit1=0  base1=MSB of Input  bit0=0  base0=LSB of Input  dbl_ovrd=off  block_type=slice
      settings: infoedit="Extracts a given range of bits from each input sample and presents it at the output.  The output type is ordinarily unsigned with binary point at zero, but can be Boolean when the slice is one bit wide.<P><P>Hardware notes: In hardware this block costs nothing."  block_version=VER_STRING_GOES_HERE
  8MSB : xbsIndex_r4/Slice  nbits=8  boolean_output=off  mode=Upper Bit Location + Width  bit1=0  base1=MSB of Input  bit0=0  base0=LSB of Input  dbl_ovrd=off  block_type=slice
      settings: infoedit="Extracts a given range of bits from each input sample and presents it at the output.  The output type is ordinarily unsigned with binary point at zero, but can be Boolean when the slice is one bit wide.<P><P>Hardware notes: In hardware this block costs nothing."  block_version=VER_STRING_GOES_HERE
  Assert : xbsIndex_r4/Assert  assert_type=off  type_source=Explicitly  gui_display_data_type=Fixed-point  arith_type="Signed  (2's comp)"  n_bits=16  bin_pt=14  preci_type=Single  exp_width=8  frac_width=24  assert_rate=on  rate_source=Explicitly  period=2  output_port=on  block_type=assert
      settings: infoedit=Asserts a user-defined sample rate and/or type on a signal.<P><P>Hardware notes: In hardware this block costs nothing.  block_version=VER_STRING_GOES_HERE
  CRC Remainders : xbsIndex_r4/ROM  depth=256  initVector=CRC_Table16  distributed_mem=Distributed memory  rst=off  init_reg=0  en=off  latency=0  gui_display_data_type=Fixed-point  arith_type=Unsigned  n_bits=16  bin_pt=0  preci_type=Single  exp_width=8  frac_width=24  dbl_ovrd=off  optimize=Area  use_rpm=off  block_type=sprom
      settings: xl_area=[0, 0, 0, 0, 0, 0, 0]  block_version=VER_STRING_GOES_HERE
  Concat : xbsIndex_r4/Concat  num_inputs=2  dbl_ovrd=off  block_type=concat
      settings: block_version=VER_STRING_GOES_HERE
  Constant : xbsIndex_r4/Constant  const=0  gui_display_data_type=Fixed-point  arith_type=Unsigned  n_bits=8  bin_pt=0  preci_type=Single  exp_width=8  frac_width=24  explicit_period=off  period=1  equ=P=C  opselect=C  inp2=PCIN>>17  opr=+  inp1=P  carry=CIN  dbl_ovrd=off  block_type=constant
      settings: block_version=VER_STRING_GOES_HERE
  Logical1 : xbsIndex_r4/Logical  logical_function=XOR  inputs=2  en=off  latency=0  precision=Full  arith_type=Unsigned  n_bits=8  bin_pt=2  align_bp=on  dbl_ovrd=off  block_type=logical
      settings: xl_area=[0, 0, 0, 0, 0, 0, 0]  block_version=VER_STRING_GOES_HERE
  Logical2 : xbsIndex_r4/Logical  logical_function=XOR  inputs=2  en=off  latency=0  precision=Full  arith_type=Unsigned  n_bits=8  bin_pt=2  align_bp=on  dbl_ovrd=off  block_type=logical
      settings: xl_area=[0, 0, 0, 0, 0, 0, 0]  block_version=VER_STRING_GOES_HERE
  crc_accum : xbsIndex_r4/Register  init=0*hex2dec('FFFF')  rst=on  en=on  dbl_ovrd=off  block_type=register
      settings: xl_area=[0, 0, 0, 0, 0, 0, 0]  block_version=VER_STRING_GOES_HERE
  16-bit CRC : Outport
  wires:
  Assert/1 -> 8LSB/1, 8MSB/1, 16-bit CRC/1
  Vin/1 -> crc_accum/3
  ByteIn/1 -> Logical1/2
  Rst/1 -> crc_accum/2
  CRC Remainders/1 -> Logical2/2
  Concat/1 -> Logical2/1
  Constant/1 -> Concat/2
  8LSB/1 -> Concat/1
  Logical2/1 -> crc_accum/1
  Logical1/1 -> CRC Remainders/1
  crc_accum/1 -> Assert/1
  8MSB/1 -> Logical1/1
